simulink model "TosAeb"  (R2021a)
settings: Solver=FixedStepDiscrete  StartTime=0.0  StopTime=inf  FixedStep=0.01  RelTol=1e-3
undefined symbols (not defined in the file or in its folder): ACCModSts, AccSts1, AEB_ObjectMovingOrientation, AEB_ObjectMovingState, CN_BTCActive, CN_CurrentGearR, CN_CurrentGearR_DHT, CN_PTCActive, CN_VDCActive, CN_VSC_EspOff, CN_VSC_FCW_AEB_Error, CN_VSC_FCW_AEB_NoDispay, CN_VSC_FCW_AEB_PerfmDgdtn, CN_VSC_FCW_AEB_TempUnAvlbl, CN_VSC_VehStandstill, CNaccelAvoidMax, CNaccelAvoidMin, CNbrakePedalPress, CNcomfortStrLeftAccelMax, CNcomfortStrLeftAccelMin, CNcomfortStrRightAccelMax, CNcomfortStrRightAccelMin, CNDrvDoorOpen, CNDrvModeEco, CNDrvModeNorm, CNDrvModeOther, CNDrvModeSport, CNDrvSBRreminder, CNNull, CNobjInvalidID, CNPassDoorOpen, CNPassSBRreminder, CNPEBSAccelNegInf, CNPEBSAccelNull, CNPEBSTimeInf, CNPEBSTimeNull, CNSTOPMAX, CNSTOPNULL, CNsubjWidth, CNSwtOFF, CNSwtON, CNTTCMAX, CNTTCNULL, CNVehConfigAEBCycNone, CNVehConfigAEBNone, CNVehConfigAEBPedNone, CNVehConfigFCWNone, CNVehConfigJANone, CollisionSeverity, FALSE, FCWSnvty, JACycObjCalcInfoDefaultParams, JAPedObjCalcInfoDefaultParams, JAVehObjCalcInfoDefaultParams, latCycObjCalcInfoDefaultParams, latPedObjCalcInfoDefaultParams, longObjCalcInfoDefaultParams, ModSts, movingStatus, ObjectType ... (+1112)
libraries: simulink x3031, AEBSFunctionLib x50, Ta_Library x33, AEBSStateMachineLib x23, Mylibrary x12, simulink_extras x7, ObjSelect_lib x3, dspstat3 x2
(part 87 of 102)

== system TosAeb/AEB_Objsel/TA_Processor/AEB_ObjSelect/TargetInfoCheck/Data_precess/TA_EffectCalculate/Obj_FilterFlgCalculate/Long_ObjFilter/TTR_Calculate/CalculateTTRAndTTM_else/LongitudinalTTRComputer/CalculateTTI/StationaryObject/TimeToIntersectionComputer ==
  note: 求解一元二次方程，并判断是否存在解、
      | 依据位置关系进行判断输出TTI数值
  note: 100
  note: -100
  note: a*t^2/2 + v*t + x = 0
  VRel : Inport  OutDataTypeStr=single
      settings: AttributesFormatString=DT=%<OutDataTypeStr>
  ARel : Inport  Port=2  OutDataTypeStr=single
      settings: AttributesFormatString=DT=%<OutDataTypeStr>
  Posn : Inport  Port=3  OutDataTypeStr=single
      settings: AttributesFormatString=DT=%<OutDataTypeStr>
  AEB_State : Inport  Port=4
  Abs : Abs
  Abs1 : Abs
  Compare To Constant : simulink/Logic and Bit Operations/Compare To Constant  relop=<=  const=0.25  OutDataTypeStr=boolean
  Compare To Constant1 : simulink/Logic and Bit Operations/Compare To Constant  relop===  const=0  OutDataTypeStr=boolean
  Compare To Constant10 : simulink/Logic and Bit Operations/Compare To Constant  relop===  const=0  OutDataTypeStr=boolean
  Compare To Constant2 : simulink/Logic and Bit Operations/Compare To Constant  relop=~=  const=0  OutDataTypeStr=boolean
  Compare To Constant3 : simulink/Logic and Bit Operations/Compare To Constant  relop===  const=0  OutDataTypeStr=boolean
  Compare To Constant4 : simulink/Logic and Bit Operations/Compare To Constant  relop=~=  const=100  OutDataTypeStr=boolean
  Compare To Constant5 : simulink/Logic and Bit Operations/Compare To Constant  relop=~=  const=-100  OutDataTypeStr=boolean
  Compare To Constant7 : simulink/Logic and Bit Operations/Compare To Constant  relop=~=  const=0  OutDataTypeStr=boolean
  Compare To Constant8 : simulink/Logic and Bit Operations/Compare To Constant  relop=<=  const=6  OutDataTypeStr=boolean
  Compare To Constant9 : simulink/Logic and Bit Operations/Compare To Constant  relop=<=  const=6  OutDataTypeStr=boolean
  Compare To Zero : Subsystem  mask(Compare To Zero){relop=<, OutDataTypeStr=boolean, ZeroCross=on}
      mask prompts: relop "Simulink:masks:Operator_MP"; OutDataTypeStr "Simulink:masks:OutputDataType_MP"; ZeroCross "Simulink:masks:EnableZerocrossingDetection_MP"
      mask help: Simulink:masks:CompareToZero_MD
      mask init: same as TosAeb/AEB_Objsel/TA_Processor/AEB_ObjSelect/TargetInfoCheck/Data_precess/TA_EffectCalculate/Obj_FilterFlgCalculate/Long_ObjFilter/TTR_Calculate/CalculateTTRAndTTM_else/ModifyTTRAtSameSpeedAndAcceleration/Compare To Zero
      mask TypeOptions relop: == | ~= | < | <= | >= | >
      mask TypeOptions OutDataTypeStr: Simulink:masks:uint8 | Simulink:masks:boolean
      mask Callback ZeroCross: sl('zeroCrossEnable', gcbh);
      mask Display: disp([relop,' 0'])
  Constant : Constant  OutDataTypeStr=single
  Constant1 : Constant  Value=P_TA_TiLowrLimInAsyEvlrCritEve  OutDataTypeStr=single
      settings: AttributesFormatString=DT=%<OutDataTypeStr>
  Constant3 : Constant  Value=P_TA_TiUpprLimInAsyEvlrCritEve  OutDataTypeStr=single
      settings: AttributesFormatString=DT=%<OutDataTypeStr>
  Delay : Delay  DelayLength=5
  Divide : Product  Inputs=*/
  Gain : Gain  Gain=0.5
  Logical Operator : Logic  Operator=OR  Inputs=3  AllPortsSameDT=off  OutDataTypeStr=boolean
  Logical Operator1 : Logic  Inputs=3  AllPortsSameDT=off  OutDataTypeStr=boolean
  Logical Operator2 : Logic  AllPortsSameDT=off  OutDataTypeStr=boolean
  Logical Operator3 : Logic  Inputs=3  AllPortsSameDT=off  OutDataTypeStr=boolean
  QuadraticEquationSolver : MATLAB Function  TreatAsAtomicUnit=on  RTWFileName=QuadraticEquationSolverNew  mask(Quadratic equation solver){ZeroThreshold=single(1e-2)}
      mask prompts: ZeroThreshold "Zero threshold:"
      mask help:
      | This block solves a quadratic polynomial equation.
      | Both solutions are returned in a bus.
      | If the equation lacks real roots then zeros are returned,
      | along with a flag indicating that no solutions where found.
      settings: RTWFcnNameOpts=Use subsystem name
  Relational Operator : RelationalOperator  Operator=<=  OutDataTypeStr=boolean  RndMeth=Simplest
  SolutionChooser : Subsystem
  Switch : Switch  Criteria=u2 > Threshold
  Switch1 : Switch  Criteria=u2 ~= 0  InputSameDT=on
      settings: AttributesFormatString=InputSameDT=%<InputSameDT>
  Switch2 : Switch  Criteria=u2 ~= 0  InputSameDT=on
      settings: AttributesFormatString=InputSameDT=%<InputSameDT>
  Switch3 : Switch  Criteria=u2 > Threshold
  Switch4 : Switch  Criteria=u2 > Threshold
  Terminator1 : Terminator
  Unit Delay : UnitDelay  SampleTime=-1  HasFrameUpgradeWarning=on
  TimeToIntersection : Outport  OutDataTypeStr=single
      settings: AttributesFormatString=DT=%<OutDataTypeStr>
  wires:
  Switch1/1 -> Switch2/3  "DefaultTTI"
  Constant1/1 -> Switch1/1  "DefaultNegativeTTI"
  Compare To Zero/1(y) -> Switch1/2
  SolutionChooser/1(Solution) -> Switch2/1
  QuadraticEquationSolver/2(SolutionFound) -> [Solution], Switch2/2
  QuadraticEquationSolver/1(x) -> SolutionChooser/1(Solutions)
  Posn/1 -> [B], Divide/1, QuadraticEquationSolver/3(c), Compare To Zero/1(u)  "Posn"
  VRel/1 -> QuadraticEquationSolver/2(b), [VRel]  "VRel"
  Switch2/1 -> Switch/3  "TimeToIntersection"
  Constant3/1 -> Switch1/3  "DefaultPositiveTTI"
  Gain/1 -> QuadraticEquationSolver/1(a)  "ARelHalf"
  [ARel] -> SolutionChooser/2(ARel)
  [ARel] -> Abs1/1
  [Solution] -> Compare To Constant1/1
  Compare To Constant1/1 -> Logical Operator/2
  Compare To Constant/1 -> Logical Operator/1
  Compare To Constant3/1 -> Switch3/2
  Constant/1 -> Switch3/1
  [VRel] -> Abs/1, Compare To Constant3/1
  Switch3/1 -> Divide/2
  Abs/1 -> Switch3/3
  Divide/1 -> [A]
  [A] -> Switch/1
  Switch4/1 -> Unit Delay/1, TimeToIntersection/1
  Abs1/1 -> Compare To Constant/1
  ARel/1 -> [ARel], Gain/1  "ARel"
  Unit Delay/1 -> Compare To Constant8/1, Compare To Constant5/1, Compare To Constant4/1, Switch4/1
  [A] -> Compare To Constant9/1, Compare To Constant7/1
  [B] -> Delay/1, Relational Operator/1
  Compare To Constant4/1 -> Logical Operator1/1
  Compare To Constant5/1 -> Logical Operator1/2
  Compare To Constant8/1 -> Logical Operator1/3
  [Solution] -> Compare To Constant10/1
  Delay/1 -> Relational Operator/2
  Compare To Constant7/1 -> Logical Operator2/1
  Compare To Constant9/1 -> Logical Operator2/2
  Logical Operator1/1 -> Logical Operator3/1
  Logical Operator2/1 -> Logical Operator3/2
  Relational Operator/1 -> Terminator1/1
  Logical Operator3/1 -> Switch4/2
  Switch/1 -> Switch4/3
  Logical Operator/1 -> Switch/2
  Compare To Constant2/1 -> Logical Operator/3
  AEB_State/1 -> Compare To Constant2/1
  Compare To Constant10/1 -> Logical Operator3/3

== system TosAeb/AEB_Objsel/TA_Processor/AEB_ObjSelect/TargetInfoCheck/Data_precess/TA_EffectCalculate/Obj_FilterFlgCalculate/Long_ObjFilter/TTR_Calculate/CalculateTTRAndTTM_else/LongitudinalTTRComputer/CalculateTTI/StationaryObject/TimeToIntersectionComputer1 ==
  note: a*t^2/2 + v*t + x = 0
  note: -100
  note: 100
  note: 求解一元二次方程，并判断是否存在解、
      | 依据位置关系进行判断输出TTI数值
  VRel : Inport  OutDataTypeStr=single
      settings: AttributesFormatString=DT=%<OutDataTypeStr>
  ARel : Inport  Port=2  OutDataTypeStr=single
      settings: AttributesFormatString=DT=%<OutDataTypeStr>
  Posn : Inport  Port=3  OutDataTypeStr=single
      settings: AttributesFormatString=DT=%<OutDataTypeStr>
  Abs : Abs
  Abs1 : Abs
  Compare To Constant : simulink/Logic and Bit Operations/Compare To Constant  relop=<=  const=0.25  OutDataTypeStr=boolean
  Compare To Constant1 : simulink/Logic and Bit Operations/Compare To Constant  relop===  const=0  OutDataTypeStr=boolean
  Compare To Constant2 : simulink/Logic and Bit Operations/Compare To Constant  relop=<  const=0  OutDataTypeStr=boolean
  Compare To Constant3 : simulink/Logic and Bit Operations/Compare To Constant  relop===  const=0  OutDataTypeStr=boolean
  Compare To Zero : Subsystem  mask(Compare To Zero){relop=<, OutDataTypeStr=boolean, ZeroCross=on}
      mask prompts: relop "Simulink:masks:Operator_MP"; OutDataTypeStr "Simulink:masks:OutputDataType_MP"; ZeroCross "Simulink:masks:EnableZerocrossingDetection_MP"
      mask help: Simulink:masks:CompareToZero_MD
      mask init: same as TosAeb/AEB_Objsel/TA_Processor/AEB_ObjSelect/TargetInfoCheck/Data_precess/TA_EffectCalculate/Obj_FilterFlgCalculate/Long_ObjFilter/TTR_Calculate/CalculateTTRAndTTM_else/ModifyTTRAtSameSpeedAndAcceleration/Compare To Zero
      mask TypeOptions relop: == | ~= | < | <= | >= | >
      mask TypeOptions OutDataTypeStr: Simulink:masks:uint8 | Simulink:masks:boolean
      mask Callback ZeroCross: sl('zeroCrossEnable', gcbh);
      mask Display: disp([relop,' 0'])
  Constant : Constant  OutDataTypeStr=single
  Constant1 : Constant  Value=P_TA_TiLowrLimInAsyEvlrCritEve  OutDataTypeStr=single
      settings: AttributesFormatString=DT=%<OutDataTypeStr>
  Constant3 : Constant  Value=P_TA_TiUpprLimInAsyEvlrCritEve  OutDataTypeStr=single
      settings: AttributesFormatString=DT=%<OutDataTypeStr>
  Divide : Product  Inputs=*/
  Gain : Gain  Gain=0.5
  Logical Operator : Logic  Inputs=3  AllPortsSameDT=off  OutDataTypeStr=boolean
  QuadraticEquationSolver : MATLAB Function  TreatAsAtomicUnit=on  RTWFileName=QuadraticEquationSolverNew  mask(Quadratic equation solver){ZeroThreshold=single(1e-2)}
      mask prompts: ZeroThreshold "Zero threshold:"
      mask help:
      | This block solves a quadratic polynomial equation.
      | Both solutions are returned in a bus.
      | If the equation lacks real roots then zeros are returned,
      | along with a flag indicating that no solutions where found.
      settings: RTWFcnNameOpts=Use subsystem name
  SolutionChooser : Subsystem
  Switch : Switch  Criteria=u2 > Threshold
  Switch1 : Switch  Criteria=u2 ~= 0  InputSameDT=on
      settings: AttributesFormatString=InputSameDT=%<InputSameDT>
  Switch2 : Switch  Criteria=u2 ~= 0  InputSameDT=on
      settings: AttributesFormatString=InputSameDT=%<InputSameDT>
  Switch3 : Switch  Criteria=u2 > Threshold
  TimeToIntersection : Outport  OutDataTypeStr=single
      settings: AttributesFormatString=DT=%<OutDataTypeStr>
  wires:
  Abs1/1 -> Compare To Constant/1
  Switch/1 -> TimeToIntersection/1
  [A] -> Switch/1
  Divide/1 -> [A]
  Abs/1 -> Switch3/3
  Switch3/1 -> Divide/2
  [VRel] -> Compare To Constant3/1, Abs/1
  Constant/1 -> Switch3/1
  Compare To Constant3/1 -> Switch3/2
  Compare To Constant2/1 -> Logical Operator/3
  [VRel] -> Compare To Constant2/1
  Logical Operator/1 -> Switch/2
  Compare To Constant1/1 -> Logical Operator/2
  Compare To Constant/1 -> Logical Operator/1
  [Solution] -> Compare To Constant1/1
  [ARel] -> Abs1/1
  [ARel] -> SolutionChooser/2(ARel)
  Gain/1 -> QuadraticEquationSolver/1(a)  "ARelHalf"
  Constant3/1 -> Switch1/3  "DefaultPositiveTTI"
  Switch2/1 -> Switch/3  "TimeToIntersection"
  VRel/1 -> [VRel], QuadraticEquationSolver/2(b)  "VRel"
  Posn/1 -> Compare To Zero/1(u), QuadraticEquationSolver/3(c), Divide/1  "Posn"
  ARel/1 -> [ARel], Gain/1  "ARel"
  QuadraticEquationSolver/1(x) -> SolutionChooser/1(Solutions)
  QuadraticEquationSolver/2(SolutionFound) -> Switch2/2, [Solution]
  SolutionChooser/1(Solution) -> Switch2/1
  Compare To Zero/1(y) -> Switch1/2
  Constant1/1 -> Switch1/1  "DefaultNegativeTTI"
  Switch1/1 -> Switch2/3  "DefaultTTI"

== system TosAeb/AEB_Objsel/TA_Processor/AEB_ObjSelect/TargetInfoCheck/Data_precess/TA_EffectCalculate/Obj_FilterFlgCalculate/Long_ObjFilter/TTR_Calculate/CalculateTTRAndTTM_else/LongitudinalTTRComputer/SelectTTI/CheckIfVehicleStops/Compare To Zero1 ==
  u : Inport
  Compare : RelationalOperator  Operator=>  OutDataTypeStr=boolean  RndMeth=Nearest  InputSameDT=on
  Constant : Constant  DisableCoverage=on  Value=0  OutDataTypeStr=Inherit: Inherit via back propagation  PreserveConstantTs=on
  y : Outport
  wires:
  Constant/1 -> Compare/2
  u/1 -> Compare/1
  Compare/1 -> y/1

== system TosAeb/AEB_Objsel/TA_Processor/AEB_ObjSelect/TargetInfoCheck/Data_precess/TA_EffectCalculate/Obj_FilterFlgCalculate/Long_ObjFilter/TTR_Calculate/CalculateTTRAndTTM_else/LongitudinalTTRComputer/SelectTTI/CheckIfVehicleStops/Compare To Zero2 ==
  u : Inport
  Compare : RelationalOperator  Operator=>  OutDataTypeStr=boolean  RndMeth=Nearest  InputSameDT=on
  Constant : Constant  DisableCoverage=on  Value=0  OutDataTypeStr=Inherit: Inherit via back propagation  PreserveConstantTs=on
  y : Outport
  wires:
  Constant/1 -> Compare/2
  u/1 -> Compare/1
  Compare/1 -> y/1

== system TosAeb/AEB_Objsel/TA_Processor/AEB_ObjSelect/TargetInfoCheck/Data_precess/TA_EffectCalculate/Obj_FilterFlgCalculate/Long_ObjFilter/TTR_Calculate/CalculateTTRAndTTM_else/LongitudinalTTRComputer/SelectTTI/CheckIfVehicleStops1/Compare To Zero1 ==
  u : Inport
  Compare : RelationalOperator  Operator=>  OutDataTypeStr=boolean  RndMeth=Nearest  InputSameDT=on
  Constant : Constant  DisableCoverage=on  Value=0  OutDataTypeStr=Inherit: Inherit via back propagation  PreserveConstantTs=on
  y : Outport
  wires:
  Constant/1 -> Compare/2
  u/1 -> Compare/1
  Compare/1 -> y/1

== system TosAeb/AEB_Objsel/TA_Processor/AEB_ObjSelect/TargetInfoCheck/Data_precess/TA_EffectCalculate/Obj_FilterFlgCalculate/Long_ObjFilter/TTR_Calculate/CalculateTTRAndTTM_else/LongitudinalTTRComputer/SelectTTI/CheckIfVehicleStops1/Compare To Zero2 ==
  u : Inport
  Compare : RelationalOperator  Operator=>  OutDataTypeStr=boolean  RndMeth=Nearest  InputSameDT=on
  Constant : Constant  DisableCoverage=on  Value=0  OutDataTypeStr=Inherit: Inherit via back propagation  PreserveConstantTs=on
  y : Outport
  wires:
  Constant/1 -> Compare/2
  u/1 -> Compare/1
  Compare/1 -> y/1

== system TosAeb/AEB_Objsel/TA_Processor/AEB_ObjSelect/TargetInfoCheck/Data_precess/TA_EffectCalculate/Obj_FilterFlgCalculate/Long_ObjFilter/TTR_Calculate/CalculateTTRAndTTM_else/LongitudinalTTRComputer/SelectTTI/HostAndObjectAreMoving/NoOperation ==
  In : Inport
      settings: AttributesFormatString=DT=%<OutDataTypeStr>
  Signal Conversion : SignalConversion  ConversionOutput=Contiguous copy  OverrideOpt=off
  Out : Outport
      settings: AttributesFormatString=DT=%<OutDataTypeStr>
  wires:
  In/1 -> Signal Conversion/1  "In"
  Signal Conversion/1 -> Out/1  "Out"

== system TosAeb/AEB_Objsel/TA_Processor/AEB_ObjSelect/TargetInfoCheck/Data_precess/TA_EffectCalculate/Obj_FilterFlgCalculate/Long_ObjFilter/TTR_Calculate/CalculateTTRAndTTM_else/LongitudinalTTRComputer/SelectTTI/HostAndObjectAreMoving/NoOperation1 ==
  In : Inport
      settings: AttributesFormatString=DT=%<OutDataTypeStr>
  Signal Conversion : SignalConversion  ConversionOutput=Contiguous copy  OverrideOpt=off
  Out : Outport
      settings: AttributesFormatString=DT=%<OutDataTypeStr>
  wires:
  In/1 -> Signal Conversion/1  "In"
  Signal Conversion/1 -> Out/1  "Out"

== system TosAeb/AEB_Objsel/TA_Processor/AEB_ObjSelect/TargetInfoCheck/Data_precess/TA_EffectCalculate/Obj_FilterFlgCalculate/Long_ObjFilter/TTR_Calculate/CalculateTTRAndTTM_else/LongitudinalTTRComputer/SelectTTI/HostOnlyWillStop/NoOperation ==
  In : Inport
      settings: AttributesFormatString=DT=%<OutDataTypeStr>
  Signal Conversion : SignalConversion  ConversionOutput=Contiguous copy  OverrideOpt=off
  Out : Outport
      settings: AttributesFormatString=DT=%<OutDataTypeStr>
  wires:
  In/1 -> Signal Conversion/1  "In"
  Signal Conversion/1 -> Out/1  "Out"

== system TosAeb/AEB_Objsel/TA_Processor/AEB_ObjSelect/TargetInfoCheck/Data_precess/TA_EffectCalculate/Obj_FilterFlgCalculate/Long_ObjFilter/TTR_Calculate/CalculateTTRAndTTM_else/LongitudinalTTRComputer/SelectTTI/HostOnlyWillStop/NoOperation1 ==
  In : Inport
      settings: AttributesFormatString=DT=%<OutDataTypeStr>
  Signal Conversion : SignalConversion  ConversionOutput=Contiguous copy  OverrideOpt=off
  Out : Outport
      settings: AttributesFormatString=DT=%<OutDataTypeStr>
  wires:
  In/1 -> Signal Conversion/1  "In"
  Signal Conversion/1 -> Out/1  "Out"

== system TosAeb/AEB_Objsel/TA_Processor/AEB_ObjSelect/TargetInfoCheck/Data_precess/TA_EffectCalculate/Obj_FilterFlgCalculate/Long_ObjFilter/TTR_Calculate/CalculateTTRAndTTM_else/LongitudinalTTRComputer/SelectTTI/ObjectOnlyWillStop/NoOperation ==
  In : Inport
      settings: AttributesFormatString=DT=%<OutDataTypeStr>
  Signal Conversion : SignalConversion  ConversionOutput=Contiguous copy  OverrideOpt=off
  Out : Outport
      settings: AttributesFormatString=DT=%<OutDataTypeStr>
  wires:
  In/1 -> Signal Conversion/1  "In"
  Signal Conversion/1 -> Out/1  "Out"

== system TosAeb/AEB_Objsel/TA_Processor/AEB_ObjSelect/TargetInfoCheck/Data_precess/TA_EffectCalculate/Obj_FilterFlgCalculate/Long_ObjFilter/TTR_Calculate/CalculateTTRAndTTM_else/LongitudinalTTRComputer/SelectTTI/ObjectOnlyWillStop/NoOperation1 ==
  In : Inport
      settings: AttributesFormatString=DT=%<OutDataTypeStr>
  Signal Conversion : SignalConversion  ConversionOutput=Contiguous copy  OverrideOpt=off
  Out : Outport
      settings: AttributesFormatString=DT=%<OutDataTypeStr>
  wires:
  In/1 -> Signal Conversion/1  "In"
  Signal Conversion/1 -> Out/1  "Out"

== system TosAeb/AEB_Objsel/TA_Processor/AEB_ObjSelect/TargetInfoCheck/Data_precess/TA_EffectCalculate/Obj_FilterFlgCalculate/Long_ObjFilter/TTR_Calculate/CalculateTTRAndTTM_else/Obj_AbsInfo/LongitudinalMotionData/Subsystem/Subsystem1 ==
  AEB : Inport
  TA_ObjAccel : Inport  Port=2
  Subj_Ax : Inport  Port=3
  MappedAngle : Inport  Port=4
  Add : Sum
  Constant : Constant  Value=0  OutDataTypeStr=single
  Product : Product
  Sin : Trigonometry  Operator=cos
  Switch : Switch  Criteria=u2 > Threshold
  Switch1 : Switch  Criteria=u2 > Threshold
  Obj_RefAcclx : Outport
  Obj_AbsAcclx : Outport  Port=2
  wires:
  MappedAngle/1 -> Sin/1
  Sin/1 -> Product/2
  TA_ObjAccel/1 -> Product/1
  Product/1 -> Switch/3, Add/1
  Switch1/1 -> Obj_AbsAcclx/1
  Subj_Ax/1 -> Add/2
  AEB/1 -> Switch1/2, Switch/2
  Constant/1 -> Switch1/1, Switch/1
  Add/1 -> Switch1/3
  Switch/1 -> Obj_RefAcclx/1

== system TosAeb/AEB_Objsel/TA_Processor/AEB_ObjSelect/TargetInfoCheck/Data_precess/TA_EffectCalculate/Obj_FilterFlgCalculate/Long_ObjFilter/TTR_Calculate/CalculateTTRAndTTM_else/Obj_AbsInfo/StoppingDataForTTICalculation/LimitedSafeDivide/SafeDivide ==
  Numerator : Inport  OutDataTypeStr=DataType
      settings: AttributesFormatString=DT=%<OutDataTypeStr>
  Denominator : Inport  Port=2  OutDataTypeStr=DataType
      settings: AttributesFormatString=DT=%<OutDataTypeStr>
  SafeDivide : MATLAB Function
  Quotient : Outport  OutDataTypeStr=DataType
      settings: AttributesFormatString=DT=%<OutDataTypeStr>
  wires:
  SafeDivide/1(Quotient) -> Quotient/1
  Denominator/1 -> SafeDivide/2(Denominator)  "Denominator"
  Numerator/1 -> SafeDivide/1(Numerator)  "Numerator"

== system TosAeb/AEB_Objsel/TA_Processor/AEB_ObjSelect/TargetInfoCheck/Data_precess/TA_EffectCalculate/Obj_FilterFlgCalculate/Long_ObjFilter/TTR_Calculate/CalculateTTRAndTTM_else/Obj_AbsInfo/StoppingDataForTTICalculation/LimitedSafeDivide/Saturation Dynamic ==
  up : Inport
  u : Inport  Port=2
  lo : Inport  Port=3
  Data Type Duplicate : DataTypeDuplicate  DisableCoverage=on
  Data Type Propagation : S-Function  FunctionName=sfix_dtprop  Parameters=14,PropDataTypeMode,(getdatatypespecs(PropDataType,PropScaling,DblOver,0, 0, PropDataTypeMode, PropScalingMode)),IfRefDouble,IfRefSingle,IsSigned,NumBitsBase,NumBitsMult,NumBitsAdd,(sort(reshape(round(NumBitsAllowFinal), prod(size(NumBitsAllowFinal)),1))),PropScalingMode,ValuesUsedBestPrec,SlopeBase,SlopeMult,SlopeAdd,BiasBase,BiasMult,BiasAdd,DblOver  SFunctionDeploymentMode=off  EnableBusSupport=off  mask(Data Type Propagation){PropDataTypeMode=Inherit via propagation rule, PropDataType=fixdt(1, 16), IfRefDouble=double, IfRefSingle=single, IsSigned=IsSigned1, NumBitsBase=NumBits1, NumBitsMult=1, NumBitsAdd=0, NumBitsAllowFinal=1:128, PropScalingMode=Inherit via propagation rule, PropScaling=2^-10, ValuesUsedBestPrec=[5 -7], SlopeBase=Slope1, SlopeMult=1, SlopeAdd=0, BiasBase=Bias1, BiasMult=1, BiasAdd=0}
      mask prompts: PropDataTypeMode "Simulink:masks:x1PropagatedDataType_MP"; PropDataType "Simulink:masks:x11PropagatedDataTypeegFixdt116Fixdtsingle_MP"; IfRefDouble "Simulink:masks:x11IfAnyReferenceInputIsDoubleOutputIs_MP"; IfRefSingle "Simulink:masks:x12IfAnyReferenceInputIsSingleOutputIs_MP"; IsSigned "Simulink:masks:x13IsSigned_MP"; NumBitsBase "Simulink:masks:x141NumberofBitsBase_MP"; NumBitsMult "Simulink:masks:x142NumberofBitsMultiplicativeAdjustment_MP"; NumBitsAdd "Simulink:masks:x143NumberofBitsAdditiveAdjustment_MP"; NumBitsAllowFinal "Simulink:masks:x144NumberofBitsAllowableFinalValues_MP"; PropScalingMode "Simulink:masks:x2PropagatedScaling_MP"; PropScaling "Simulink:masks:x21PropagatedScalingSlopeEg29OrSlopeBiasEg1253_MP"; ValuesUsedBestPrec "Simulink:masks:x21ValuesUsedToDetermineBestPrecisionScaling_MP"; SlopeBase "Simulink:masks:x211SlopeBase_MP"; SlopeMult "Simulink:masks:x212SlopeMultiplicativeAdjustment_MP"; SlopeAdd "Simulink:masks:x213SlopeAdditiveAdjustment_MP"; BiasBase "Simulink:masks:x221BiasBase_MP"; BiasMult "Simulink:masks:x222BiasMultiplicativeAdjustment_MP"; BiasAdd "Simulink:masks:x223BiasAdditiveAdjustment_MP"
      mask help: Simulink:masks:DataTypePropagation_MD
      mask init:
      | DblOver = 0;
      mask TypeOptions PropDataTypeMode: Simulink:masks:SpecifyViaDialog | Simulink:masks:InheritViaPropagationRule
      mask Callback PropDataTypeMode: fixptdialog(gcbh)
      mask Callback PropDataType: fixptdialog(gcbh)
      mask TypeOptions IfRefDouble: Simulink:masks:double | Simulink:masks:single
      mask TypeOptions IfRefSingle: Simulink:masks:double | Simulink:masks:single
      mask TypeOptions IsSigned: Simulink:masks:IsSigned1 | Simulink:masks:IsSigned2 | Simulink:masks:IsSigned1OrIsSigned2 | Simulink:masks:TRUE | Simulink:masks:FALSE
      mask TypeOptions NumBitsBase: NumBits1 | NumBits2 | max([NumBits1 NumBits2]) | min([NumBits1 NumBits2]) | NumBits1+NumBits2
      mask TypeOptions PropScalingMode: Simulink:masks:SpecifyViaDialog | Simulink:masks:InheritViaPropagationRule | Simulink:masks:ObtainViaBestPrecision
      mask Callback PropScalingMode: fixptdialog(gcbh)
      mask TypeOptions SlopeBase: Slope1 | Slope2 | max([Slope1 Slope2]) | min([Slope1 Slope2]) | Slope1*Slope2 | Slope1/Slope2 | PosRange1 | PosRange2 | max([PosRange1 PosRange2]) | min([PosRange1 PosRange2]) | PosRange1*PosRange2 | PosRange1/PosRange2
      mask TypeOptions BiasBase: Bias1 | Bias2 | max([Bias1 Bias2]) | min([Bias1 Bias2]) | Bias1*Bias2 | Bias1/Bias2 | Bias1+Bias2 | Bias1-Bias2
      mask Display:
      | port_label('Input',1,'Ref1');
      | port_label('Input',2,'Ref2');
      | port_label('Input',3,'Prop');
  LowerRelop1 : RelationalOperator  DisableCoverage=on  Operator=>  OutDataTypeStr=boolean  RndMeth=Nearest
  Switch : Switch  Criteria=u2 ~= 0  OutDataTypeStr=Inherit: Inherit via back propagation  ZeroCross=off
  Switch2 : Switch  Criteria=u2 ~= 0  OutMin=OutMin  OutMax=OutMax  OutDataTypeStr=Inherit: Inherit via back propagation  ZeroCross=off
  UpperRelop : RelationalOperator  DisableCoverage=on  Operator=<  OutDataTypeStr=boolean  RndMeth=Nearest
  y : Outport  OutMin=OutMin  OutMax=OutMax
  wires:
  up/1 -> Switch2/1, LowerRelop1/2
  u/1 -> Data Type Propagation/2, Data Type Propagation/1, LowerRelop1/1, Switch/3, UpperRelop/1
  lo/1 -> Switch/1, UpperRelop/2
  Switch/1 -> Data Type Duplicate/2, Switch2/3, Data Type Propagation/3
  Switch2/1 -> Data Type Duplicate/1, y/1
  LowerRelop1/1 -> Switch2/2
  UpperRelop/1 -> Switch/2

== system TosAeb/AEB_Objsel/TA_Processor/AEB_ObjSelect/TargetInfoCheck/Data_precess/TA_EffectCalculate/Obj_FilterFlgCalculate/Long_ObjFilter/TTR_Calculate/CalculateTTRAndTTM_else/Obj_AbsInfo/StoppingDataForTTICalculation/LimitedSafeDivide1/SafeDivide ==
  Numerator : Inport  OutDataTypeStr=DataType
      settings: AttributesFormatString=DT=%<OutDataTypeStr>
  Denominator : Inport  Port=2  OutDataTypeStr=DataType
      settings: AttributesFormatString=DT=%<OutDataTypeStr>
  SafeDivide : MATLAB Function
  Quotient : Outport  OutDataTypeStr=DataType
      settings: AttributesFormatString=DT=%<OutDataTypeStr>
  wires:
  SafeDivide/1(Quotient) -> Quotient/1
  Denominator/1 -> SafeDivide/2(Denominator)  "Denominator"
  Numerator/1 -> SafeDivide/1(Numerator)  "Numerator"

== system TosAeb/AEB_Objsel/TA_Processor/AEB_ObjSelect/TargetInfoCheck/Data_precess/TA_EffectCalculate/Obj_FilterFlgCalculate/Long_ObjFilter/TTR_Calculate/CalculateTTRAndTTM_else/Obj_AbsInfo/StoppingDataForTTICalculation/LimitedSafeDivide1/Saturation Dynamic ==
  up : Inport
  u : Inport  Port=2
  lo : Inport  Port=3
  Data Type Duplicate : DataTypeDuplicate  DisableCoverage=on
  Data Type Propagation : S-Function  FunctionName=sfix_dtprop  Parameters=14,PropDataTypeMode,(getdatatypespecs(PropDataType,PropScaling,DblOver,0, 0, PropDataTypeMode, PropScalingMode)),IfRefDouble,IfRefSingle,IsSigned,NumBitsBase,NumBitsMult,NumBitsAdd,(sort(reshape(round(NumBitsAllowFinal), prod(size(NumBitsAllowFinal)),1))),PropScalingMode,ValuesUsedBestPrec,SlopeBase,SlopeMult,SlopeAdd,BiasBase,BiasMult,BiasAdd,DblOver  SFunctionDeploymentMode=off  EnableBusSupport=off  mask(Data Type Propagation){PropDataTypeMode=Inherit via propagation rule, PropDataType=fixdt(1, 16), IfRefDouble=double, IfRefSingle=single, IsSigned=IsSigned1, NumBitsBase=NumBits1, NumBitsMult=1, NumBitsAdd=0, NumBitsAllowFinal=1:128, PropScalingMode=Inherit via propagation rule, PropScaling=2^-10, ValuesUsedBestPrec=[5 -7], SlopeBase=Slope1, SlopeMult=1, SlopeAdd=0, BiasBase=Bias1, BiasMult=1, BiasAdd=0}
      mask prompts: PropDataTypeMode "Simulink:masks:x1PropagatedDataType_MP"; PropDataType "Simulink:masks:x11PropagatedDataTypeegFixdt116Fixdtsingle_MP"; IfRefDouble "Simulink:masks:x11IfAnyReferenceInputIsDoubleOutputIs_MP"; IfRefSingle "Simulink:masks:x12IfAnyReferenceInputIsSingleOutputIs_MP"; IsSigned "Simulink:masks:x13IsSigned_MP"; NumBitsBase "Simulink:masks:x141NumberofBitsBase_MP"; NumBitsMult "Simulink:masks:x142NumberofBitsMultiplicativeAdjustment_MP"; NumBitsAdd "Simulink:masks:x143NumberofBitsAdditiveAdjustment_MP"; NumBitsAllowFinal "Simulink:masks:x144NumberofBitsAllowableFinalValues_MP"; PropScalingMode "Simulink:masks:x2PropagatedScaling_MP"; PropScaling "Simulink:masks:x21PropagatedScalingSlopeEg29OrSlopeBiasEg1253_MP"; ValuesUsedBestPrec "Simulink:masks:x21ValuesUsedToDetermineBestPrecisionScaling_MP"; SlopeBase "Simulink:masks:x211SlopeBase_MP"; SlopeMult "Simulink:masks:x212SlopeMultiplicativeAdjustment_MP"; SlopeAdd "Simulink:masks:x213SlopeAdditiveAdjustment_MP"; BiasBase "Simulink:masks:x221BiasBase_MP"; BiasMult "Simulink:masks:x222BiasMultiplicativeAdjustment_MP"; BiasAdd "Simulink:masks:x223BiasAdditiveAdjustment_MP"
      mask help: Simulink:masks:DataTypePropagation_MD
      mask init:
      | DblOver = 0;
      mask TypeOptions PropDataTypeMode: Simulink:masks:SpecifyViaDialog | Simulink:masks:InheritViaPropagationRule
      mask Callback PropDataTypeMode: fixptdialog(gcbh)
      mask Callback PropDataType: fixptdialog(gcbh)
      mask TypeOptions IfRefDouble: Simulink:masks:double | Simulink:masks:single
      mask TypeOptions IfRefSingle: Simulink:masks:double | Simulink:masks:single
      mask TypeOptions IsSigned: Simulink:masks:IsSigned1 | Simulink:masks:IsSigned2 | Simulink:masks:IsSigned1OrIsSigned2 | Simulink:masks:TRUE | Simulink:masks:FALSE
      mask TypeOptions NumBitsBase: NumBits1 | NumBits2 | max([NumBits1 NumBits2]) | min([NumBits1 NumBits2]) | NumBits1+NumBits2
      mask TypeOptions PropScalingMode: Simulink:masks:SpecifyViaDialog | Simulink:masks:InheritViaPropagationRule | Simulink:masks:ObtainViaBestPrecision
      mask Callback PropScalingMode: fixptdialog(gcbh)
      mask TypeOptions SlopeBase: Slope1 | Slope2 | max([Slope1 Slope2]) | min([Slope1 Slope2]) | Slope1*Slope2 | Slope1/Slope2 | PosRange1 | PosRange2 | max([PosRange1 PosRange2]) | min([PosRange1 PosRange2]) | PosRange1*PosRange2 | PosRange1/PosRange2
      mask TypeOptions BiasBase: Bias1 | Bias2 | max([Bias1 Bias2]) | min([Bias1 Bias2]) | Bias1*Bias2 | Bias1/Bias2 | Bias1+Bias2 | Bias1-Bias2
      mask Display:
      | port_label('Input',1,'Ref1');
      | port_label('Input',2,'Ref2');
      | port_label('Input',3,'Prop');
  LowerRelop1 : RelationalOperator  DisableCoverage=on  Operator=>  OutDataTypeStr=boolean  RndMeth=Nearest
  Switch : Switch  Criteria=u2 ~= 0  OutDataTypeStr=Inherit: Inherit via back propagation  ZeroCross=off
  Switch2 : Switch  Criteria=u2 ~= 0  OutMin=OutMin  OutMax=OutMax  OutDataTypeStr=Inherit: Inherit via back propagation  ZeroCross=off
  UpperRelop : RelationalOperator  DisableCoverage=on  Operator=<  OutDataTypeStr=boolean  RndMeth=Nearest
  y : Outport  OutMin=OutMin  OutMax=OutMax
  wires:
  up/1 -> Switch2/1, LowerRelop1/2
  u/1 -> Data Type Propagation/2, Data Type Propagation/1, LowerRelop1/1, Switch/3, UpperRelop/1
  lo/1 -> Switch/1, UpperRelop/2
  Switch/1 -> Data Type Duplicate/2, Switch2/3, Data Type Propagation/3
  Switch2/1 -> Data Type Duplicate/1, y/1
  LowerRelop1/1 -> Switch2/2
  UpperRelop/1 -> Switch/2

== system TosAeb/AEB_Objsel/TA_Processor/AEB_ObjSelect/TargetInfoCheck/Data_precess/TA_EffectCalculate/ValideateObj/ObjPos_Calculate/ObjPos_Calculateelse/InPath_Cal/DetermineInPath/PredictHostLateralPositionAtTTR/CalculateHeading/CheckSpeed ==
  x : Inport
  y : Inport  Port=2
  LatSpdThresholdNonVeh1 : Constant  Value=P_TA_SpdThdInAsyEvlrCritEve
  Product : Product
  Product1 : Product
  Product2 : Product
  Relational Operator : RelationalOperator  Operator=>  OutDataTypeStr=boolean  RndMeth=Nearest
  Subtract : Sum
  SpeedValid : Outport
  wires:
  y/1 -> Product/1, Product/2
  Subtract/1 -> Relational Operator/1  "VelocitySquared"
  Product1/1 -> Subtract/1
  Product/1 -> Subtract/2
  x/1 -> Product1/2, Product1/1
  LatSpdThresholdNonVeh1/1 -> Product2/1, Product2/2
  Product2/1 -> Relational Operator/2
  Relational Operator/1 -> SpeedValid/1  "SpeedValid"

== system TosAeb/AEB_Objsel/TA_Processor/AEB_ObjSelect/TargetInfoCheck/Data_precess/TA_EffectCalculate/ValideateObj/ObjPos_Calculate/ObjPos_Calculateelse/InPath_Cal/DetermineInPath/PredictHostLateralPositionAtTTR/CalculateHeading/ExtractVelocitiesAtStartOfSegments ==
  PathEstimn : Inport  OutDataTypeStr=Bus: PahEstimn
  Assignment : Assignment  OutputInitialize=Specify size for each dimension in table  IndexOptions=Index vector (port)  Indices=1  OutputSizes=4
  Assignment1 : Assignment  OutputInitialize=Specify size for each dimension in table  IndexOptions=Index vector (port)  Indices=1  OutputSizes=4
  Assignment2 : Assignment  OutputInitialize=Specify size for each dimension in table  IndexOptions=Index vector (port)  Indices=1  OutputSizes=4
  CheckSpeed : Subsystem
  ExtractVelocityVectorFirstPoint : Subsystem
  For Iterator : ForIterator  ResetStates=reset  IterationLimit=4  IterationVariableDataType=int8
  SpeedValid : Outport
  SegmentVelocitiesX : Outport  Port=2
  SegmentVelocitiesY : Outport  Port=3
  wires:
  Assignment2/1 -> SegmentVelocitiesY/1  "SegmentVelocitiesY"
  Assignment1/1 -> SegmentVelocitiesX/1  "SegmentVelocitiesX"
  Assignment/1 -> SpeedValid/1  "SpeedValid"
  CheckSpeed/1(SpeedValid) -> Assignment/1
  [Index] -> Assignment/2, Assignment1/2, Assignment2/2
  ExtractVelocityVectorFirstPoint/2(dy) -> Assignment2/1, CheckSpeed/2(y)
  ExtractVelocityVectorFirstPoint/1(dx) -> Assignment1/1, CheckSpeed/1(x)
  For Iterator/1 -> [Index]
  PathEstimn/1 -> ExtractVelocityVectorFirstPoint/1(PathEstimn)

== system TosAeb/AEB_Objsel/TA_Processor/AEB_ObjSelect/TargetInfoCheck/Data_precess/TA_EffectCalculate/ValideateObj/ObjPos_Calculate/ObjPos_Calculateelse/InPath_Cal/DetermineInPath/PredictHostLateralPositionAtTTR/SegmentComponents/SafeDivide ==
  Numerator : Inport  OutDataTypeStr=DataType
      settings: AttributesFormatString=DT=%<OutDataTypeStr>
  Denominator : Inport  Port=2  OutDataTypeStr=DataType
      settings: AttributesFormatString=DT=%<OutDataTypeStr>
  SafeDivide : MATLAB Function
  Quotient : Outport  OutDataTypeStr=DataType
      settings: AttributesFormatString=DT=%<OutDataTypeStr>
  wires:
  SafeDivide/1(Quotient) -> Quotient/1
  Denominator/1 -> SafeDivide/2(Denominator)  "Denominator"
  Numerator/1 -> SafeDivide/1(Numerator)  "Numerator"

== system TosAeb/AEB_Objsel/TA_Processor/AEB_ObjSelect/TargetInfoCheck/Data_precess/TA_EffectCalculate/ValideateObj/ObjPos_Calculate/ObjPos_Calculateelse/InPath_Cal/DetermineInPath/PredictHostLateralPositionAtTTR/SelectSegment/ForEachSegmentInPahEstimn ==
  PahEstimn : Inport
  Time : Inport  Port=2
  Assignment5 : Assignment  OutputInitialize=Specify size for each dimension in table  IndexOptions=Index vector (port)  Indices=1  OutputSizes=4
  Bus Selector : BusSelector  OutputSignals=TiAry
  Compare To Constant : simulink/Logic and Bit Operations/Compare To Constant  relop===  const=4  OutDataTypeStr=boolean
  Constant : Constant  OutDataTypeStr=boolean
  For Iterator : ForIterator  IterationLimit=4
  Logical Operator : Logic  AllPortsSameDT=off  OutDataTypeStr=boolean
  Logical Operator1 : Logic  AllPortsSameDT=off  OutDataTypeStr=boolean
  Min : MinMax  Function=max  OutDataTypeStr=single
  Min1 : MinMax  OutDataTypeStr=single
  Relational Operator : RelationalOperator  Operator=>  OutDataTypeStr=boolean  RndMeth=Nearest  InputSameDT=on
      settings: AttributesFormatString=InputSameDT=%<InputSameDT>
  Relational Operator1 : RelationalOperator  Operator=<=  OutDataTypeStr=boolean  RndMeth=Nearest  InputSameDT=on
      settings: AttributesFormatString=InputSameDT=%<InputSameDT>
  Relational Operator2 : RelationalOperator  Operator=>  OutDataTypeStr=boolean  RndMeth=Nearest  InputSameDT=on
      settings: AttributesFormatString=InputSameDT=%<InputSameDT>
  Selector : Selector  InputPortWidth=4  IndexOptions=Index vector (port)  Indices=[1 3]  OutputSizes=1
  Switch : Switch  Criteria=u2 ~= 0
  TimeWithinSegment : Outport
  wires:
  Min/1 -> [A], Relational Operator1/2
  Time/1 -> [Time], Relational Operator1/1
  Min1/1 -> Relational Operator/2
  [Time] -> Relational Operator/1
  Relational Operator1/1 -> Logical Operator/1
  Relational Operator/1 -> Logical Operator/2
  PahEstimn/1 -> Selector/1
  For Iterator/1 -> [Index]
  Bus Selector/1 -> Min/1, Min1/1  "<TiAry>"
  [Index] -> Compare To Constant/1, Selector/2
  Assignment5/1 -> TimeWithinSegment/1  "TimeWithinSegment"
  [Index] -> Assignment5/2
  Selector/1 -> Bus Selector/1
  [Time] -> Relational Operator2/1
  [A] -> Relational Operator2/2
  Compare To Constant/1 -> Logical Operator1/1
  Relational Operator2/1 -> Logical Operator1/2
  Logical Operator/1 -> Switch/3
  Switch/1 -> Assignment5/1
  Logical Operator1/1 -> [Valid]
  [Valid] -> Switch/2
  Constant/1 -> Switch/1

== system TosAeb/FcwAebJa_Step_sys/ALG_AEBSystem/ALG_AEBController/AEB_LongTgtInfoCalc/LongObjCalcInfoCalc/AEBS_LongCollisionAssesApp/LongCollisionAvoidAssess/HighLvlBrkCalc/HighLvlBrkCalc4Brake/accelAvoidHighLvlBrkSituation2/accelAvoidHighLvlBrkCalc/If Action Subsystem1/accelAvoidLowLvlBrkCalc/Situation1 ==
  dxEgoBrake : Inport
  vx : Inport  Port=2
  tObjStop : Inport  Port=3
  subjVel : Inport  Port=4
  vxAbsObj : Inport  Port=5
  axAbsObj : Inport  Port=6
  function : TriggerPort  TriggerType=function-call  VariantControl=Variant
  Bus Creator : BusCreator  Inputs=6
  Bus Selector : BusSelector  OutputSignals=dxEgoBrake,vx,tObjStop
  Bus Selector1 : BusSelector  OutputSignals=axAbsObj,vx,dxEgoBrake
  Bus Selector2 : BusSelector  OutputSignals=subjVel,vxAbsObj,axAbsObj,dxEgoBrake
  Case1 : Subsystem  TreatAsAtomicUnit=on
  Case2 : Subsystem  TreatAsAtomicUnit=on
  If : If  IfExpression=u1
  Merge : Merge
  collisionFirstFlgCalc : Subsystem
  accelAvoidLowLvlBrk : Outport
  wires:
  If/1 -> Case1/Action
  Merge/1 -> accelAvoidLowLvlBrk/1
  Case1/1(accelAvoid) -> Merge/1
  Case2/1(accelAvoid) -> Merge/2
  If/2 -> [CASE2]
  [CASE2] -> Case2/Action
  tObjStop/1 -> Bus Creator/3  "tObjStop"
  vx/1 -> Bus Creator/2  "vx"
  subjVel/1 -> Bus Creator/4  "subjVel"
  dxEgoBrake/1 -> Bus Creator/1  "dxEgoBrake"
  axAbsObj/1 -> Bus Creator/6  "axAbsObj"
  vxAbsObj/1 -> Bus Creator/5  "vxAbsObj"
  Bus Creator/1 -> Bus Selector2/1, Bus Selector1/1, Bus Selector/1
  Bus Selector1/1 -> Case1/1(axAbsObj)  "<axAbsObj>"
  Bus Selector1/2 -> Case1/2(vx)  "<vx>"
  Bus Selector1/3 -> Case1/3(dxEgoBrake)  "<dxEgoBrake>"
  Bus Selector2/1 -> Case2/1(subjVel)  "<subjVel>"
  Bus Selector2/2 -> Case2/2(vxAbsObj)  "<vxAbsObj>"
  Bus Selector2/3 -> Case2/3(axAbsObj)  "<axAbsObj>"
  Bus Selector2/4 -> Case2/4(dxEgoBrake)  "<dxEgoBrake>"
  Bus Selector/1 -> collisionFirstFlgCalc/1(dxEgoBrake)  "<dxEgoBrake>"
  Bus Selector/2 -> collisionFirstFlgCalc/2(vx)  "<vx>"
  Bus Selector/3 -> collisionFirstFlgCalc/3(tObjStop)  "<tObjStop>"
  collisionFirstFlgCalc/1(collisionFirstFlg) -> If/1

== system TosAeb/FcwAebJa_Step_sys/ALG_AEBSystem/ALG_AEBController/AEB_LongTgtInfoCalc/LongObjCalcInfoCalc/AEBS_LongCollisionAssesApp/LongCollisionAvoidAssess/HighLvlBrkCalc/HighLvlBrkCalc4Brake/accelAvoidHighLvlBrkSituation2/accelAvoidHighLvlBrkCalc/If Action Subsystem1/accelAvoidLowLvlBrkCalc/Situation2 ==
  axAbsObj : Inport
  vx : Inport  Port=2
  dxEgoBrake : Inport  Port=3
  function : TriggerPort  TriggerType=function-call  VariantControl=Variant
  Divide : Product  Inputs=*/
  Gain : Gain  Gain=2
  Math Function : Math  Operator=square  SignedPower=on  SaturateOnIntegerOverflow=on
  Subtract : Sum  Inputs=+-
  accelAvoidLowLvlBrk : Outport
  wires:
  axAbsObj/1 -> Subtract/1
  vx/1 -> Math Function/1
  Math Function/1 -> Divide/1
  dxEgoBrake/1 -> Gain/1
  Gain/1 -> Divide/2
  Subtract/1 -> accelAvoidLowLvlBrk/1
  Divide/1 -> Subtract/2

== system TosAeb/FcwAebJa_Step_sys/ALG_AEBSystem/ALG_AEBController/AEB_LongTgtInfoCalc/LongObjCalcInfoCalc/AEBS_LongCollisionAssesApp/LongCollisionAvoidAssess/HighLvlBrkCalc/HighLvlBrkCalc4Brake/accelAvoidHighLvlBrkSituation2/accelAvoidHighLvlBrkCalc/If Action Subsystem1/accelAvoidLowLvlBrkCalc/Situation3 ==
  note: aEgo^2
      | aAvoid = ----------------------------------
      | 		vObj^2
      | 		---------  - 2(dRel - dScure)
      | 		aObj
  subjVel : Inport
  vxAbsObj : Inport  Port=2
  axAbsObj : Inport  Port=3
  dxEgoBrake : Inport  Port=4
  function : TriggerPort  TriggerType=function-call  VariantControl=Variant
  Divide : Product  Inputs=*/
  Divide1 : Product  Inputs=*/
  Gain : Gain  Gain=2
  Math Function : Math  Operator=square  SignedPower=on  SaturateOnIntegerOverflow=on
  Math Function1 : Math  Operator=square  SignedPower=on  SaturateOnIntegerOverflow=on
  Subtract : Sum  Inputs=+-
  accelAvoidLowLvlBrk : Outport
  wires:
  vxAbsObj/1 -> Math Function/1
  subjVel/1 -> Math Function1/1
  Math Function/1 -> Divide/1
  Gain/1 -> Subtract/2
  Divide1/1 -> accelAvoidLowLvlBrk/1
  Math Function1/1 -> Divide1/1
  Divide/1 -> Subtract/1
  axAbsObj/1 -> Divide/2
  dxEgoBrake/1 -> Gain/1
  Subtract/1 -> Divide1/2

== system TosAeb/FcwAebJa_Step_sys/ALG_AEBSystem/ALG_AEBController/AEB_LongTgtInfoCalc/LongObjCalcInfoCalc/AEBS_LongCollisionAssesApp/LongCollisionAvoidAssess/HighLvlBrkCalc/HighLvlBrkCalc4Brake/accelAvoidHighLvlBrkSituation2/accelAvoidHighLvlBrkCalc/If Action Subsystem1/accelAvoidLowLvlBrkCalc/Situation4 ==
  function : TriggerPort  TriggerType=function-call  VariantControl=Variant
  Constant : Constant  Value=CNNull  VectorParams1D=off
  accelAvoidLowLvlBrk : Outport
  wires:
  Constant/1 -> accelAvoidLowLvlBrk/1

== system TosAeb/FcwAebJa_Step_sys/ALG_AEBSystem/ALG_AEBController/AEB_LongTgtInfoCalc/LongObjCalcInfoCalc/AEBS_LongCollisionAssesApp/LongCollisionAvoidAssess/HighLvlBrkCalc/HighLvlBrkCalc4Brake/accelAvoidHighLvlBrkSituation2/accelAvoidHighLvlBrkCalc/If Action Subsystem1/accelAvoidLowLvlBrkCalc/accelAvoidLowLvlBrkLimit ==
  accelAvoidLowLvlBrkNoLimit : Inport
  Saturation : Saturate  UpperLimit=CNNull  LowerLimit=CNaccelAvoidMin
      settings: AttributesFormatString=|
      | UpperLimit:%<UpperLimit>
      | LowerLimit:%<LowerLimit>
  accelAvoidLowLvlBrk : Outport
  wires:
  accelAvoidLowLvlBrkNoLimit/1 -> Saturation/1
  Saturation/1 -> accelAvoidLowLvlBrk/1

== system TosAeb/FcwAebJa_Step_sys/ALG_AEBSystem/ALG_AEBController/AEB_LongTgtInfoCalc/LongObjCalcInfoCalc/AEBS_LongCollisionAssesApp/LongCollisionAvoidAssess/HighLvlBrkCalc/HighLvlBrkCalc4Brake/accelAvoidHighLvlBrkSituation2/accelAvoidHighLvlBrkCalc/If Action Subsystem1/accelAvoidLowLvlBrkCalc1/Situation1 ==
  dxEgoBrake : Inport
  vx : Inport  Port=2
  tObjStop : Inport  Port=3
  subjVel : Inport  Port=4
  vxAbsObj : Inport  Port=5
  axAbsObj : Inport  Port=6
  function : TriggerPort  TriggerType=function-call  VariantControl=Variant
  Bus Creator : BusCreator  Inputs=6
  Bus Selector : BusSelector  OutputSignals=dxEgoBrake,vxRelTargetObjCalc,tObjStop
  Bus Selector1 : BusSelector  OutputSignals=axAbsTargetObjCalc,vxRelTargetObjCalc,dxEgoBrake
  Bus Selector2 : BusSelector  OutputSignals=subjVel,vxAbsTargetObjCalc,axAbsTargetObjCalc,dxEgoBrake
  Case1 : Subsystem  TreatAsAtomicUnit=on
  Case2 : Subsystem  TreatAsAtomicUnit=on
  If : If  IfExpression=u1
  Merge : Merge
  collisionFirstFlgCalc : Subsystem
  accelAvoidLowLvlBrk : Outport
  wires:
  collisionFirstFlgCalc/1(collisionFirstFlg) -> If/1
  Bus Selector/3 -> collisionFirstFlgCalc/3(tObjStop)  "<tObjStop>"
  Bus Selector/2 -> collisionFirstFlgCalc/2(vx)  "<vxRelTargetObjCalc>"
  Bus Selector/1 -> collisionFirstFlgCalc/1(dxEgoBrake)  "<dxEgoBrake>"
  Bus Selector2/4 -> Case2/4(dxEgoBrake)  "<dxEgoBrake>"
  Bus Selector2/3 -> Case2/3(axAbsObj)  "<axAbsTargetObjCalc>"
  Bus Selector2/2 -> Case2/2(vxAbsObj)  "<vxAbsTargetObjCalc>"
  Bus Selector2/1 -> Case2/1(subjVel)  "<subjVel>"
  Bus Selector1/3 -> Case1/3(dxEgoBrake)  "<dxEgoBrake>"
  Bus Selector1/2 -> Case1/2(vx)  "<vxRelTargetObjCalc>"
  Bus Selector1/1 -> Case1/1(axAbsObj)  "<axAbsTargetObjCalc>"
  Bus Creator/1 -> Bus Selector/1, Bus Selector1/1, Bus Selector2/1
  vxAbsObj/1 -> Bus Creator/5  "vxAbsTargetObjCalc"
  axAbsObj/1 -> Bus Creator/6  "axAbsTargetObjCalc"
  dxEgoBrake/1 -> Bus Creator/1  "dxEgoBrake"
  subjVel/1 -> Bus Creator/4  "subjVel"
  vx/1 -> Bus Creator/2  "vxRelTargetObjCalc"
  tObjStop/1 -> Bus Creator/3  "tObjStop"
  [CASE2] -> Case2/Action
  If/2 -> [CASE2]
  Case2/1(accelAvoid) -> Merge/2
  Case1/1(accelAvoid) -> Merge/1
  Merge/1 -> accelAvoidLowLvlBrk/1
  If/1 -> Case1/Action

== system TosAeb/FcwAebJa_Step_sys/ALG_AEBSystem/ALG_AEBController/AEB_LongTgtInfoCalc/LongObjCalcInfoCalc/AEBS_LongCollisionAssesApp/LongCollisionAvoidAssess/HighLvlBrkCalc/HighLvlBrkCalc4Brake/accelAvoidHighLvlBrkSituation2/accelAvoidHighLvlBrkCalc/If Action Subsystem1/accelAvoidLowLvlBrkCalc1/Situation2 ==
  axAbsObj : Inport
  vx : Inport  Port=2
  dxEgoBrake : Inport  Port=3
  function : TriggerPort  TriggerType=function-call  VariantControl=Variant
  Divide : Product  Inputs=*/
  Gain : Gain  Gain=2
  Math Function : Math  Operator=square  SignedPower=on  SaturateOnIntegerOverflow=on
  Subtract : Sum  Inputs=+-
  accelAvoidLowLvlBrk : Outport
  wires:
  Divide/1 -> Subtract/2
  Subtract/1 -> accelAvoidLowLvlBrk/1
  Gain/1 -> Divide/2
  dxEgoBrake/1 -> Gain/1
  Math Function/1 -> Divide/1
  vx/1 -> Math Function/1
  axAbsObj/1 -> Subtract/1

== system TosAeb/FcwAebJa_Step_sys/ALG_AEBSystem/ALG_AEBController/AEB_LongTgtInfoCalc/LongObjCalcInfoCalc/AEBS_LongCollisionAssesApp/LongCollisionAvoidAssess/HighLvlBrkCalc/HighLvlBrkCalc4Brake/accelAvoidHighLvlBrkSituation2/accelAvoidHighLvlBrkCalc/If Action Subsystem1/accelAvoidLowLvlBrkCalc1/Situation3 ==
  note: aEgo^2
      | aAvoid = ----------------------------------
      | 		vObj^2
      | 		---------  - 2(dRel - dScure)
      | 		aObj
  subjVel : Inport
  vxAbsObj : Inport  Port=2
  axAbsObj : Inport  Port=3
  dxEgoBrake : Inport  Port=4
  function : TriggerPort  TriggerType=function-call  VariantControl=Variant
  Divide : Product  Inputs=*/
  Divide1 : Product  Inputs=*/
  Gain : Gain  Gain=2
  Math Function : Math  Operator=square  SignedPower=on  SaturateOnIntegerOverflow=on
  Math Function1 : Math  Operator=square  SignedPower=on  SaturateOnIntegerOverflow=on
  Subtract : Sum  Inputs=+-
  accelAvoidLowLvlBrk : Outport
  wires:
  Subtract/1 -> Divide1/2
  dxEgoBrake/1 -> Gain/1
  axAbsObj/1 -> Divide/2
  Divide/1 -> Subtract/1
  Math Function1/1 -> Divide1/1
  Divide1/1 -> accelAvoidLowLvlBrk/1
  Gain/1 -> Subtract/2
  Math Function/1 -> Divide/1
  subjVel/1 -> Math Function1/1
  vxAbsObj/1 -> Math Function/1

== system TosAeb/FcwAebJa_Step_sys/ALG_AEBSystem/ALG_AEBController/AEB_LongTgtInfoCalc/LongObjCalcInfoCalc/AEBS_LongCollisionAssesApp/LongCollisionAvoidAssess/HighLvlBrkCalc/HighLvlBrkCalc4Brake/accelAvoidHighLvlBrkSituation2/accelAvoidHighLvlBrkCalc/If Action Subsystem1/accelAvoidLowLvlBrkCalc1/Situation4 ==
  function : TriggerPort  TriggerType=function-call  VariantControl=Variant
  Constant : Constant  Value=CNaccelAvoidMax  VectorParams1D=off
  accelAvoidLowLvlBrk : Outport
  wires:
  Constant/1 -> accelAvoidLowLvlBrk/1

== system TosAeb/FcwAebJa_Step_sys/ALG_AEBSystem/ALG_AEBController/AEB_LongTgtInfoCalc/LongObjCalcInfoCalc/AEBS_LongCollisionAssesApp/LongCollisionAvoidAssess/HighLvlBrkCalc/HighLvlBrkCalc4Brake/accelAvoidHighLvlBrkSituation2/accelAvoidHighLvlBrkCalc/If Action Subsystem1/accelAvoidLowLvlBrkCalc1/accelAvoidLowLvlBrkLimit ==
  accelAvoidLowLvlBrkNoLimit : Inport
  Saturation : Saturate  UpperLimit=CNaccelAvoidMax  LowerLimit=CNaccelAvoidMin
      settings: AttributesFormatString=|
      | UpperLimit:%<UpperLimit>
      | LowerLimit:%<LowerLimit>
  accelAvoidLowLvlBrk : Outport
  wires:
  Saturation/1 -> accelAvoidLowLvlBrk/1
  accelAvoidLowLvlBrkNoLimit/1 -> Saturation/1

== system TosAeb/FcwAebJa_Step_sys/ALG_AEBSystem/ALG_AEBController/AEB_LongTgtInfoCalc/LongObjCalcInfoCalc/AEBS_LongCollisionAssesApp/LongCollisionAvoidAssess/HighLvlBrkCalc/HighLvlBrkCalc4Brake/accelAvoidHighLvlBrkSituation3/If Action Subsystem1/accelAvoidHighLvlBrkCalc/accelAvoidLowLvlBrkCalc/Situation1 ==
  dxEgoBrake : Inport
  vx : Inport  Port=2
  tObjStop : Inport  Port=3
  subjVel : Inport  Port=4
  vxAbsObj : Inport  Port=5
  axAbsObj : Inport  Port=6
  function : TriggerPort  TriggerType=function-call  VariantControl=Variant
  Bus Creator : BusCreator  Inputs=6
  Bus Selector : BusSelector  OutputSignals=dxEgoBrake,vx,tObjStop
  Bus Selector1 : BusSelector  OutputSignals=axAbsObj,vx,dxEgoBrake
  Bus Selector2 : BusSelector  OutputSignals=subjVel,vxAbsObj,axAbsObj,dxEgoBrake
  Case1 : Subsystem  TreatAsAtomicUnit=on
  Case2 : Subsystem  TreatAsAtomicUnit=on
  If : If  IfExpression=u1
  Merge : Merge
  collisionFirstFlgCalc : Subsystem
  accelAvoidLowLvlBrk : Outport
  wires:
  If/1 -> Case1/Action
  Merge/1 -> accelAvoidLowLvlBrk/1
  Case1/1(accelAvoid) -> Merge/1
  Case2/1(accelAvoid) -> Merge/2
  If/2 -> [CASE2]
  [CASE2] -> Case2/Action
  tObjStop/1 -> Bus Creator/3  "tObjStop"
  vx/1 -> Bus Creator/2  "vx"
  subjVel/1 -> Bus Creator/4  "subjVel"
  dxEgoBrake/1 -> Bus Creator/1  "dxEgoBrake"
  axAbsObj/1 -> Bus Creator/6  "axAbsObj"
  vxAbsObj/1 -> Bus Creator/5  "vxAbsObj"
  Bus Creator/1 -> Bus Selector2/1, Bus Selector1/1, Bus Selector/1
  Bus Selector1/1 -> Case1/1(axAbsObj)  "<axAbsObj>"
  Bus Selector1/2 -> Case1/2(vx)  "<vx>"
  Bus Selector1/3 -> Case1/3(dxEgoBrake)  "<dxEgoBrake>"
  Bus Selector2/1 -> Case2/1(subjVel)  "<subjVel>"
  Bus Selector2/2 -> Case2/2(vxAbsObj)  "<vxAbsObj>"
  Bus Selector2/3 -> Case2/3(axAbsObj)  "<axAbsObj>"
  Bus Selector2/4 -> Case2/4(dxEgoBrake)  "<dxEgoBrake>"
  Bus Selector/1 -> collisionFirstFlgCalc/1(dxEgoBrake)  "<dxEgoBrake>"
  Bus Selector/2 -> collisionFirstFlgCalc/2(vx)  "<vx>"
  Bus Selector/3 -> collisionFirstFlgCalc/3(tObjStop)  "<tObjStop>"
  collisionFirstFlgCalc/1(collisionFirstFlg) -> If/1

== system TosAeb/FcwAebJa_Step_sys/ALG_AEBSystem/ALG_AEBController/AEB_LongTgtInfoCalc/LongObjCalcInfoCalc/AEBS_LongCollisionAssesApp/LongCollisionAvoidAssess/HighLvlBrkCalc/HighLvlBrkCalc4Brake/accelAvoidHighLvlBrkSituation3/If Action Subsystem1/accelAvoidHighLvlBrkCalc/accelAvoidLowLvlBrkCalc/Situation2 ==
  axAbsObj : Inport
  vx : Inport  Port=2
  dxEgoBrake : Inport  Port=3
  function : TriggerPort  TriggerType=function-call  VariantControl=Variant
  Divide : Product  Inputs=*/
  Gain : Gain  Gain=2
  Math Function : Math  Operator=square  SignedPower=on  SaturateOnIntegerOverflow=on
  Subtract : Sum  Inputs=+-
  accelAvoidLowLvlBrk : Outport
  wires:
  axAbsObj/1 -> Subtract/1
  vx/1 -> Math Function/1
  Math Function/1 -> Divide/1
  dxEgoBrake/1 -> Gain/1
  Gain/1 -> Divide/2
  Subtract/1 -> accelAvoidLowLvlBrk/1
  Divide/1 -> Subtract/2
